simulink model "TosAeb"  (R2021a)
settings: Solver=FixedStepDiscrete  StartTime=0.0  StopTime=inf  FixedStep=0.01  RelTol=1e-3
undefined symbols (not defined in the file or in its folder): ACCModSts, AccSts1, AEB_ObjectMovingOrientation, AEB_ObjectMovingState, CN_BTCActive, CN_CurrentGearR, CN_CurrentGearR_DHT, CN_PTCActive, CN_VDCActive, CN_VSC_EspOff, CN_VSC_FCW_AEB_Error, CN_VSC_FCW_AEB_NoDispay, CN_VSC_FCW_AEB_PerfmDgdtn, CN_VSC_FCW_AEB_TempUnAvlbl, CN_VSC_VehStandstill, CNaccelAvoidMax, CNaccelAvoidMin, CNbrakePedalPress, CNcomfortStrLeftAccelMax, CNcomfortStrLeftAccelMin, CNcomfortStrRightAccelMax, CNcomfortStrRightAccelMin, CNDrvDoorOpen, CNDrvModeEco, CNDrvModeNorm, CNDrvModeOther, CNDrvModeSport, CNDrvSBRreminder, CNNull, CNobjInvalidID, CNPassDoorOpen, CNPassSBRreminder, CNPEBSAccelNegInf, CNPEBSAccelNull, CNPEBSTimeInf, CNPEBSTimeNull, CNSTOPMAX, CNSTOPNULL, CNsubjWidth, CNSwtOFF, CNSwtON, CNTTCMAX, CNTTCNULL, CNVehConfigAEBCycNone, CNVehConfigAEBNone, CNVehConfigAEBPedNone, CNVehConfigFCWNone, CNVehConfigJANone, CollisionSeverity, FALSE, FCWSnvty, JACycObjCalcInfoDefaultParams, JAPedObjCalcInfoDefaultParams, JAVehObjCalcInfoDefaultParams, latCycObjCalcInfoDefaultParams, latPedObjCalcInfoDefaultParams, longObjCalcInfoDefaultParams, ModSts, movingStatus, ObjectType ... (+1112)
libraries: simulink x3031, AEBSFunctionLib x50, Ta_Library x33, AEBSStateMachineLib x23, Mylibrary x12, simulink_extras x7, ObjSelect_lib x3, dspstat3 x2
(part 18 of 102)

== system TosAeb/FcwAebJa_Step_sys/ALG_AEBSystem/ALG_AEBController/AEB_StateMachineCalcApp/VehAEBStateMachine/EBAStateMachine ==
  inputSignal : Inport
  AEBFuncFaultMap : Subsystem
  AEBStateMachine : AEBSStateMachineLib/AEBStateMachine  SystemSampleTime=-1  VariantActivationTime=update diagram  Latency=0  AutoFrameSizeCalculation=off
  ActivationCondCalc : Subsystem
  ActiveStateAborCondCalc : Subsystem
  Bus Selector : BusSelector  OutputSignals=AVHStsActv,vehStateCalcInfo.VSC_FCW_AEB_FctSts.FCW_AEB_VehFctSts,vehStateCalcInfo.VSC_AEBFuncSwtSts.AEB_VehResp,vehStateCalcInfo.VSCVehStandStill
  Bus Selector1 : BusSelector  OutputSignals=VehFuncSuppAborCond.vehEBASuppCond,vehFctStsArbSuppAborCond.brkSuppAborCondArb.EBASuppArb,FuncImpSuppAborCond.brkFuncImpSuppAborCond.brkFuncImpSuppCond.vehEBAFuncImpSuppCond
  Bus Selector2 : BusSelector  OutputSignals=VehFuncSuppAborCond.vehEBAAborCondNoStandstill,vehFctStsArbSuppAborCond.brkSuppAborCondArb.EBAAborArb,FuncImpSuppAborCond.brkFuncImpSuppAborCond.brkFuncImpAborCond.vehEBAFuncImpAborCond,FuncManeuAborCond.vehBrkManeuAborCond
  Bus Selector3 : BusSelector  OutputSignals=VehFuncSuppAborCond.vehEBAAborCondStandstill,vehFctStsArbSuppAborCond.brkSuppAborCondArb.EBAAborArb,FuncImpSuppAborCond.brkFuncImpSuppAborCond.brkFuncImpAborCond.vehEBAFuncImpAborCond,FuncManeuAborCond.vehBrkManeuAborCond
  Constant : Constant  Value=P_AEB_FuncStatus_VehEBAWorkTime
  Constant1 : Constant  Value=P_AEB_FuncStatus_VehEBAStandstillWorkTime
  Constant2 : Constant  Value=Ts
  StandstillAborCondCalc : Subsystem
  Terminator1 : Terminator
  vehEBARawModSts : Outport
  vehEBAModSts : Outport  Port=2
  wires:
  ActiveStateAborCondCalc/1(ActiveStateAborCondFulfilled) -> AEBStateMachine/6
  StandstillAborCondCalc/1(StandstillAborCondFulfilled) -> AEBStateMachine/7
  inputSignal/1 -> Bus Selector3/1, Bus Selector2/1, Bus Selector1/1, Bus Selector/1
  Bus Selector1/3 -> ActivationCondCalc/3(vehEBAFuncImpSuppCond)  "<vehEBAFuncImpSuppCond>"
  Bus Selector1/2 -> ActivationCondCalc/2(EBASuppArb)  "<EBASuppArb>"
  Bus Selector1/1 -> ActivationCondCalc/1(vehEBASuppCond)  "<vehEBASuppCond>"
  Bus Selector2/1 -> ActiveStateAborCondCalc/1(EBAAborCondNoStandstill)  "<vehEBAAborCondNoStandstill>"
  Bus Selector2/2 -> ActiveStateAborCondCalc/2(EBAAborArb)  "<EBAAborArb>"
  Bus Selector2/3 -> ActiveStateAborCondCalc/3(vehEBAFuncImpAborCond)  "<vehEBAFuncImpAborCond>"
  Bus Selector2/4 -> ActiveStateAborCondCalc/4(vehBrkManeuAborCond)  "<vehBrkManeuAborCond>"
  Bus Selector3/1 -> StandstillAborCondCalc/1(EBAAborCondStandstill)  "<vehEBAAborCondStandstill>"
  Bus Selector3/2 -> StandstillAborCondCalc/2(EBAAborArb)  "<EBAAborArb>"
  Bus Selector3/3 -> StandstillAborCondCalc/3(vehEBAFuncImpAborCond)  "<vehEBAFuncImpAborCond>"
  Bus Selector3/4 -> StandstillAborCondCalc/4(vehBrkManeuAborCond)  "<vehBrkManeuAborCond>"
  AEBStateMachine/1 -> vehEBARawModSts/1
  AEBStateMachine/2 -> vehEBAModSts/1  "vehEBAModSts"
  AEBStateMachine/3 -> Terminator1/1
  ActivationCondCalc/1(ActivationCondFulfilled) -> AEBStateMachine/5
  Constant2/1 -> AEBStateMachine/8
  Constant/1 -> AEBStateMachine/9
  Constant1/1 -> AEBStateMachine/10
  Bus Selector/2 -> AEBFuncFaultMap/1(FCW_AEB_VehFctSts)  "<FCW_AEB_VehFctSts>"
  Bus Selector/3 -> AEBStateMachine/3  "<AEB_VehResp>"
  Bus Selector/1 -> AEBStateMachine/1  "<AVHStsActv>"
  Bus Selector/4 -> AEBStateMachine/4  "<VSCVehStandStill>"
  AEBFuncFaultMap/1(Fault) -> AEBStateMachine/2

== system TosAeb/FcwAebJa_Step_sys/ALG_AEBSystem/ALG_AEBController/AEB_StateMachineCalcApp/VehAEBStateMachine/LatentWarningStateMachine ==
  inputSignal : Inport
  AEBFuncFaultMap : Subsystem
  AEBStateMachine : AEBSStateMachineLib/AEBStateMachine  SystemSampleTime=-1  VariantActivationTime=update diagram  Latency=0  AutoFrameSizeCalculation=off
  ActivationCondCalc : Subsystem
  ActiveStateAborCondCalc : Subsystem
  Bus Selector : BusSelector  OutputSignals=vehStateCalcInfo.VSC_FCW_AEB_FctSts.FCW_AEB_VehFctSts,vehStateCalcInfo.VSC_AEBFuncSwtSts.AEB_VehResp
  Bus Selector1 : BusSelector  OutputSignals=VehFuncSuppAborCond.vehLatentWarningSuppCond,vehFctStsArbSuppAborCond.warningSuppAborCond.latentWarningSuppArb,FuncImpSuppAborCond.warningFuncImpSuppAborCond.warningFuncImpSuppCond.vehLatentWarningFuncImpSuppCond
  Bus Selector2 : BusSelector  OutputSignals=VehFuncSuppAborCond.vehLatentWarningAborCond,vehFctStsArbSuppAborCond.warningSuppAborCond.latentWarningAborArb
  Constant : Constant  Value=P_AEB_FuncStatus_VehLatentWarningTime
  Constant1 : Constant  Value=P_AEB_FuncStatus_VehLatentWarningStandstillWorkTime
  Constant2 : Constant  Value=Ts
  Constant3 : Constant  Value=FALSE
  Constant4 : Constant  Value=FALSE
  Constant5 : Constant  Value=FALSE
  Terminator1 : Terminator
  vehLatentWarningRawModSts : Outport
  vehLatentWarningModSts : Outport  Port=2
  wires:
  ActiveStateAborCondCalc/1(ActiveStateAborCondFulfilled) -> AEBStateMachine/6
  ActivationCondCalc/1(ActivationCondFulfilled) -> AEBStateMachine/5
  inputSignal/1 -> Bus Selector/1, Bus Selector2/1, Bus Selector1/1
  Bus Selector1/2 -> ActivationCondCalc/2(latentWarningSuppArb)  "<latentWarningSuppArb>"
  Bus Selector1/3 -> ActivationCondCalc/3(vehLatentWarningFuncImpSuppCond)  "<vehLatentWarningFuncImpSuppCond>"
  Bus Selector1/1 -> ActivationCondCalc/1(vehLatentWarningSuppCond)  "<vehLatentWarningSuppCond>"
  Bus Selector2/1 -> ActiveStateAborCondCalc/1(vehLatentWarningAborCond)  "<vehLatentWarningAborCond>"
  Bus Selector2/2 -> ActiveStateAborCondCalc/2(latentWarningAbor)  "<latentWarningAborArb>"
  AEBStateMachine/2 -> vehLatentWarningModSts/1  "vehLatentWarningModSts"
  AEBStateMachine/1 -> vehLatentWarningRawModSts/1
  AEBStateMachine/3 -> Terminator1/1
  Constant3/1 -> AEBStateMachine/7
  Constant2/1 -> AEBStateMachine/8
  Constant/1 -> AEBStateMachine/9
  Constant1/1 -> AEBStateMachine/10
  Constant5/1 -> AEBStateMachine/1
  Constant4/1 -> AEBStateMachine/4
  Bus Selector/1 -> AEBFuncFaultMap/1(FCW_AEB_VehFctSts)  "<FCW_AEB_VehFctSts>"
  Bus Selector/2 -> AEBStateMachine/3  "<AEB_VehResp>"
  AEBFuncFaultMap/1(Fault) -> AEBStateMachine/2

== system TosAeb/FcwAebJa_Step_sys/ALG_AEBSystem/ALG_AEBController/AEB_StateMachineCalcApp/VehAEBStateMachine/PreWarningStateMachine ==
  inputSignal : Inport
  AEBFuncFaultMap : Subsystem
  AEBStateMachine : AEBSStateMachineLib/AEBStateMachine  SystemSampleTime=-1  VariantActivationTime=update diagram  Latency=0  AutoFrameSizeCalculation=off
  ActivationCondCalc : Subsystem
  ActiveStateAborCondCalc : Subsystem
  Bus Selector : BusSelector  OutputSignals=vehStateCalcInfo.VSC_FCW_AEB_FctSts.FCW_AEB_VehFctSts,vehStateCalcInfo.VSC_AEBFuncSwtSts.AEB_VehResp
  Bus Selector1 : BusSelector  OutputSignals=VehFuncSuppAborCond.vehPreWarningSuppCond,vehFctStsArbSuppAborCond.warningSuppAborCond.preWarningSuppArb,FuncImpSuppAborCond.warningFuncImpSuppAborCond.warningFuncImpSuppCond.vehPreWarningFuncImpSuppCond
  Bus Selector2 : BusSelector  OutputSignals=VehFuncSuppAborCond.vehPreWarningAborCond,vehFctStsArbSuppAborCond.warningSuppAborCond.preWarningAborArb,FuncImpSuppAborCond.warningFuncImpSuppAborCond.warningFuncImpAborCond.vehPreWarningFuncImpAborCond
  Constant : Constant  Value=P_AEB_FuncStatus_VehPreWarningWorkTime
  Constant1 : Constant  Value=P_AEB_FuncStatus_VehPreWarningStandstillWorkTime
  Constant2 : Constant  Value=Ts
  Constant3 : Constant  Value=FALSE
  Constant4 : Constant  Value=FALSE
  Constant5 : Constant  Value=FALSE
  Terminator1 : Terminator
  vehPreWarningRawModSts : Outport
  vehPreWarningModSts : Outport  Port=2
  wires:
  AEBStateMachine/2 -> vehPreWarningModSts/1  "vehPreWarningModSts"
  inputSignal/1 -> Bus Selector1/1, Bus Selector2/1, Bus Selector/1
  Bus Selector1/2 -> ActivationCondCalc/2(preWarningSuppArb)  "<preWarningSuppArb>"
  Bus Selector1/3 -> ActivationCondCalc/3(vehPreWarningFuncImpSuppCond)  "<vehPreWarningFuncImpSuppCond>"
  Bus Selector1/1 -> ActivationCondCalc/1(vehPreWarningSuppCond)  "<vehPreWarningSuppCond>"
  Bus Selector2/1 -> ActiveStateAborCondCalc/1(vehPreWarningAborCond)  "<vehPreWarningAborCond>"
  Bus Selector2/2 -> ActiveStateAborCondCalc/2(preWarningSuppArb)  "<preWarningAborArb>"
  Bus Selector2/3 -> ActiveStateAborCondCalc/3(vehPreWarningFuncImpAborCond)  "<vehPreWarningFuncImpAborCond>"
  AEBStateMachine/1 -> vehPreWarningRawModSts/1
  AEBStateMachine/3 -> Terminator1/1
  Constant5/1 -> AEBStateMachine/1
  Bus Selector/1 -> AEBFuncFaultMap/1(FCW_AEB_VehFctSts)  "<FCW_AEB_VehFctSts>"
  Bus Selector/2 -> AEBStateMachine/3  "<AEB_VehResp>"
  Constant4/1 -> AEBStateMachine/4
  ActivationCondCalc/1(vehPreWarningActivationCondFulfilled) -> AEBStateMachine/5
  ActiveStateAborCondCalc/1(vehPreWarningAborCondFulfilled) -> AEBStateMachine/6
  Constant3/1 -> AEBStateMachine/7
  Constant2/1 -> AEBStateMachine/8
  Constant/1 -> AEBStateMachine/9
  Constant1/1 -> AEBStateMachine/10
  AEBFuncFaultMap/1(Fault) -> AEBStateMachine/2

== system TosAeb/FcwAebJa_Step_sys/ALG_AEBSystem/ALG_AEBController/AEB_StateMachineCalcApp/VehAEBStateMachine/PrefillStateMachine ==
  inputSignal : Inport
  AEBFuncFaultMap : Subsystem
  AEBStateMachine : AEBSStateMachineLib/AEBStateMachine  SystemSampleTime=-1  VariantActivationTime=update diagram  Latency=0  AutoFrameSizeCalculation=off
  ActivationCondCalc : Subsystem
  ActiveStateAborCondCalc : Subsystem
  Bus Selector : BusSelector  OutputSignals=vehStateCalcInfo.VSC_FCW_AEB_FctSts.FCW_AEB_VehFctSts,vehStateCalcInfo.VSC_AEBFuncSwtSts.AEB_VehResp
  Bus Selector1 : BusSelector  OutputSignals=VehFuncSuppAborCond.vehPrefillSuppCond,FuncImpSuppAborCond.brkReadyFuncImpSuppAborCond.brkReadyFuncImpSuppCond.vehPrefillFuncImpSuppCond,vehFctStsArbSuppAborCond.brkReadySuppAborCond.prefillBrkReadySuppArb
  Bus Selector2 : BusSelector  OutputSignals=VehFuncSuppAborCond.vehPrefillAborCond,vehFctStsArbSuppAborCond.brkReadySuppAborCond.prefillBrkReadyAborArb,FuncImpSuppAborCond.brkReadyFuncImpSuppAborCond.brkReadyFuncImpAborCond.vehPrefillFuncImpAborCond,FuncManeuAborCond.ABPFuncManeuAborCond
  Constant : Constant  Value=P_AEB_FuncStatus_VehPrefillWorkTime
  Constant1 : Constant  Value=P_AEB_FuncStatus_VehPrefillStandstillWorkTime
  Constant2 : Constant  Value=Ts
  Constant3 : Constant  Value=FALSE
  Constant4 : Constant  Value=FALSE
  Constant5 : Constant  Value=FALSE
  Terminator1 : Terminator
  vehPrefillRawModSts : Outport
  vehPrefillModSts : Outport  Port=2
  wires:
  inputSignal/1 -> Bus Selector2/1, Bus Selector1/1, Bus Selector/1
  Bus Selector1/2 -> ActivationCondCalc/2(vehPrefillFuncImpSuppCond)  "<vehPrefillFuncImpSuppCond>"
  Bus Selector1/3 -> ActivationCondCalc/3(vehPrefillBrkReadySuppArb)  "<prefillBrkReadySuppArb>"
  Bus Selector1/1 -> ActivationCondCalc/1(vehPrefillSuppCond)  "<vehPrefillSuppCond>"
  Bus Selector2/1 -> ActiveStateAborCondCalc/1(vehPrefillAborCond)  "<vehPrefillAborCond>"
  Bus Selector2/2 -> ActiveStateAborCondCalc/2(prefillBrkReadyAborArb)  "<prefillBrkReadyAborArb>"
  Bus Selector2/3 -> ActiveStateAborCondCalc/3(vehPrefillFuncImpAborCond)  "<vehPrefillFuncImpAborCond>"
  Bus Selector2/4 -> ActiveStateAborCondCalc/4(brkImpOpcAborCond)  "<ABPFuncManeuAborCond>"
  AEBStateMachine/3 -> Terminator1/1
  AEBStateMachine/1 -> vehPrefillRawModSts/1
  AEBStateMachine/2 -> vehPrefillModSts/1  "vehPrefillModSts"
  Constant5/1 -> AEBStateMachine/1
  Bus Selector/1 -> AEBFuncFaultMap/1(FCW_AEB_VehFctSts)  "<FCW_AEB_VehFctSts>"
  Bus Selector/2 -> AEBStateMachine/3  "<AEB_VehResp>"
  Constant4/1 -> AEBStateMachine/4
  ActivationCondCalc/1(ActivationCondFulfilled) -> AEBStateMachine/5
  ActiveStateAborCondCalc/1(ActiveStateAborCondFulfilled) -> AEBStateMachine/6
  Constant3/1 -> AEBStateMachine/7
  Constant2/1 -> AEBStateMachine/8
  Constant/1 -> AEBStateMachine/9
  Constant1/1 -> AEBStateMachine/10
  AEBFuncFaultMap/1(Fault) -> AEBStateMachine/2

== system TosAeb/FcwAebJa_Step_sys/ALG_AEBSystem/ALG_AEBController/AEB_StateMachineCalcApp/VehFCTModStsArb/BrkArb ==
  vehAEBModSts : Inport
  BrkAborArb : Subsystem
  BrkActvArb : Subsystem
  BrkSuppArb : Subsystem
  Bus Creator : BusCreator  Inputs=6
  WarningModStsArbtrtn : Subsystem
  brkActvCond : Outport
  AEBpartModStsArb : Outport  Port=2
  AEBfullModStsArb : Outport  Port=3
  EBAModStsArb : Outport  Port=4
  brkSuppAborCondArb : Outport  Port=5
  wires:
  [AEBfullModStsArb] -> AEBfullModStsArb/1
  [AEBpartModStsArb] -> AEBpartModStsArb/1
  WarningModStsArbtrtn/3(EBAModStsArb) -> EBAModStsArb/1, BrkActvArb/3(EBAModStsArbtrtn)
  BrkAborArb/3(EBAAborArb) -> [EBAAborArb], Bus Creator/6  "EBAAborArb"
  [AEBfullAborArb] -> WarningModStsArbtrtn/3(AEBfullAborArb)
  BrkSuppArb/2(AEBfullSuppArb) -> Bus Creator/2  "AEBfullSuppArb"
  BrkActvArb/1(brkActvCond) -> brkActvCond/1
  BrkAborArb/2(AEBfullAborArb) -> [AEBfullAborArb], Bus Creator/5  "AEBfullAborArb"
  vehAEBModSts/1 -> BrkAborArb/1(vehAEBModSts), BrkSuppArb/1(vehAEBModSts), WarningModStsArbtrtn/1(vehAEBModSts)
  BrkSuppArb/3(EBASuppArb) -> Bus Creator/3  "EBASuppArb"
  Bus Creator/1 -> brkSuppAborCondArb/1
  BrkAborArb/1(AEBpartAborArb) -> [AEBpartAborArb], Bus Creator/4  "AEBpartAborArb"
  [AEBpartAborArb] -> WarningModStsArbtrtn/2(AEBpartAborArb)
  [EBAAborArb] -> WarningModStsArbtrtn/4(EBAAborArb)
  BrkSuppArb/1(AEBpartSuppArb) -> Bus Creator/1  "AEBpartSuppArb"
  WarningModStsArbtrtn/1(AEBpartModStsArb) -> [AEBpartModStsArb], BrkActvArb/1(AEBpartModStsArbtrtn)
  WarningModStsArbtrtn/2(AEBfullModStsArb) -> [AEBfullModStsArb], BrkActvArb/2(AEBfullModStsArbtrtn)

== system TosAeb/FcwAebJa_Step_sys/ALG_AEBSystem/ALG_AEBController/AEB_StateMachineCalcApp/VehFCTModStsArb/BrkReadyArb ==
  VehAEBModSts : Inport
  brkActvCond : Inport  Port=2
  BrkReadyAborArb : Subsystem
  BrkReadyActvArb : Subsystem
  BrkReadyModStsArb : Subsystem
  BrkReadySuppArb : Subsystem
  Bus Creator : BusCreator  Inputs=4
  brkReadyActvCond : Outport
  prefillModStsArb : Outport  Port=2
  ABAModStsArb : Outport  Port=3
  brkReadySuppAborCond : Outport  Port=4
  wires:
  VehAEBModSts/1 -> BrkReadyModStsArb/1(vehAEBModSts)
  brkActvCond/1 -> BrkReadyAborArb/1(brkActvCond), BrkReadySuppArb/1(brkActvCond)
  BrkReadyAborArb/1(prefillBrkReadyAborArb) -> Bus Creator/3, [prefillBrkReadyAbor]  "prefillBrkReadyAborArb"
  BrkReadyAborArb/2(ABABrkReadyAborArb) -> [ABABrkReadyAbor], Bus Creator/4  "ABABrkReadyAborArb"
  Bus Creator/1 -> brkReadySuppAborCond/1
  [ABABrkReadyAbor] -> BrkReadyModStsArb/3(ABABrkReadyAbor)
  [prefillBrkReadyAbor] -> BrkReadyModStsArb/2(prefillBrkReadyAbor)
  BrkReadyActvArb/1(brkReadyActv) -> brkReadyActvCond/1
  BrkReadySuppArb/2(ABABrkReadySuppArb) -> Bus Creator/2  "ABABrkReadySuppArb"
  BrkReadySuppArb/1(prefillBrkReadySuppArb) -> Bus Creator/1  "prefillBrkReadySuppArb"
  BrkReadyModStsArb/1(prefillModStsArb) -> prefillModStsArb/1, BrkReadyActvArb/1(prefillModStsArb)
  BrkReadyModStsArb/2(ABAModStsArb) -> ABAModStsArb/1, BrkReadyActvArb/2(ABAModStsArb)

== system TosAeb/FcwAebJa_Step_sys/ALG_AEBSystem/ALG_AEBController/AEB_StateMachineCalcApp/VehFCTModStsArb/WarningArb ==
  vehAEBModSts : Inport
  brkReadyActvCond : Inport  Port=2
  brkActvCond : Inport  Port=3
  BrkReadyAborArb : Subsystem
  Bus Creator : BusCreator  Inputs=6
  Terminator : Terminator
  WarningActvArbtrtn : Subsystem
  WarningModStsArbtrtn : Subsystem
  WarningSuppArb : Subsystem
  latentWarningModStsArb : Outport
  preWarningModStsArb : Outport  Port=2
  acuteWarningModStsArb : Outport  Port=3
  warningSuppAborCond : Outport  Port=4
  wires:
  Bus Creator/1 -> warningSuppAborCond/1
  vehAEBModSts/1 -> WarningModStsArbtrtn/1(vehAEBModSts), WarningSuppArb/1(vehAEBModSts), BrkReadyAborArb/3(vehAEBModSts)
  brkActvCond/1 -> WarningSuppArb/3(brkActvCond), BrkReadyAborArb/1(brkActvCond)
  brkReadyActvCond/1 -> WarningSuppArb/2(brkReadyActvCond), BrkReadyAborArb/2(brkReadyActvCond)
  WarningSuppArb/3(acutewarningSuppArb) -> Bus Creator/3  "acuteWarningSuppArb"
  WarningSuppArb/2(prewarningSuppArb) -> Bus Creator/2  "preWarningSuppArb"
  WarningSuppArb/1(latentWarningSuppArb) -> Bus Creator/1  "latentWarningSuppArb"
  BrkReadyAborArb/1(latentWarningAborArb) -> [latentWarningAborArb], Bus Creator/4  "latentWarningAborArb"
  BrkReadyAborArb/2(preWarningAborArb) -> [preWarningAborArb], Bus Creator/5  "preWarningAborArb"
  WarningModStsArbtrtn/1(latentWarningModStsArb) -> WarningActvArbtrtn/1(latentWarningModStsArb), [latentWarningModStsArb]
  WarningModStsArbtrtn/2(preWarningModStsArb) -> [preWarningModStsArb], WarningActvArbtrtn/2(preWarningModStsArb)
  WarningModStsArbtrtn/3(acuteWarningModStsArb) -> acuteWarningModStsArb/1, WarningActvArbtrtn/3(acuteWarningModStsArb)
  BrkReadyAborArb/3(acuteWarningAborArb) -> [acuteWarningAborArb], Bus Creator/6  "acuteWarningAborArb"
  [latentWarningAborArb] -> WarningModStsArbtrtn/2(latentWarningAborArb)
  [acuteWarningAborArb] -> WarningModStsArbtrtn/4(acuteWarningAborArb)
  [preWarningAborArb] -> WarningModStsArbtrtn/3(preWarningAborArb)
  [latentWarningModStsArb] -> latentWarningModStsArb/1
  [preWarningModStsArb] -> preWarningModStsArb/1
  WarningActvArbtrtn/1(WarningActvCond) -> Terminator/1

== system TosAeb/FcwAebJa_Step_sys/ALG_AEBSystem/ALG_AEBController/AEB_VehicleStateCalcApp/CycSuppAborCondtionCalc/CycAEBfullSuppAborConditonCalc ==
  VSCAEB_PedResp : Inport
  FCW_AEB_PedFctSts : Inport  Port=2
  vehUnstableStateFlg : Inport  Port=3
  vehAssociatedSysStateSuppCond : Inport  Port=4
  "vehConfigAEBCyc " : Inport  Port=5
  blockTimeBrakeReady : Inport  Port=6
  Bus Creator : BusCreator  Inputs=6
  Bus Selector : BusSelector  OutputSignals=AEB_PedResp,FCW_AEB_PedFctSts,vehUnstableSts,vehAssociatedSysStsSuppFlg,VehicleConfigAEBCyc,blockTimeBrake
  Bus Selector1 : BusSelector  OutputSignals=AEB_PedResp,FCW_AEB_PedFctSts,vehAssociatedSysStsSuppFlg,vehUnstableSts
  VehStateCalcCycAEBfullAborCalc : Subsystem
  VehStateCalcCycAEBfullSuppCalc : Subsystem
  VSCCycAEBfullSuppCond : Outport
  VSCCycAEBfullAborCond : Outport  Port=2
  wires:
  VehStateCalcCycAEBfullAborCalc/1(VSCCycAEBfullAborCond) -> VSCCycAEBfullAborCond/1
  VehStateCalcCycAEBfullSuppCalc/1(VSCCycAEBfullSuppCond) -> VSCCycAEBfullSuppCond/1
  vehUnstableStateFlg/1 -> Bus Creator/3
  FCW_AEB_PedFctSts/1 -> Bus Creator/2
  vehAssociatedSysStateSuppCond/1 -> Bus Creator/4
  VSCAEB_PedResp/1 -> Bus Creator/1
  "vehConfigAEBCyc "/1 -> Bus Creator/5
  blockTimeBrakeReady/1 -> Bus Creator/6
  Bus Creator/1 -> Bus Selector/1, Bus Selector1/1
  Bus Selector/4 -> VehStateCalcCycAEBfullSuppCalc/4(vehAssociatedSysStateSuppCond)  "<vehAssociatedSysStsSuppFlg>"
  Bus Selector/3 -> VehStateCalcCycAEBfullSuppCalc/3(vehUnstableStateFlg)  "<vehUnstableSts>"
  Bus Selector/5 -> VehStateCalcCycAEBfullSuppCalc/5("vehConfigAEBCyc ")  "<VehicleConfigAEBCyc>"
  Bus Selector/2 -> VehStateCalcCycAEBfullSuppCalc/2(FCW_AEB_PedFctSts)  "<FCW_AEB_PedFctSts>"
  Bus Selector/6 -> VehStateCalcCycAEBfullSuppCalc/6(blockTimeBrakeReady)  "<blockTimeBrake>"
  Bus Selector/1 -> VehStateCalcCycAEBfullSuppCalc/1(VSCAEB_PedResp)  "<AEB_PedResp>"
  Bus Selector1/1 -> VehStateCalcCycAEBfullAborCalc/1(VSCAEB_PedResp)  "<AEB_PedResp>"
  Bus Selector1/2 -> VehStateCalcCycAEBfullAborCalc/2(FCW_AEB_PedFctSts)  "<FCW_AEB_PedFctSts>"
  Bus Selector1/3 -> VehStateCalcCycAEBfullAborCalc/3(vehAssociatedSysStateSuppCond)  "<vehAssociatedSysStsSuppFlg>"
  Bus Selector1/4 -> VehStateCalcCycAEBfullAborCalc/4(vehUnstableStateFlg)  "<vehUnstableSts>"

== system TosAeb/FcwAebJa_Step_sys/ALG_AEBSystem/ALG_AEBController/AEB_VehicleStateCalcApp/CycSuppAborCondtionCalc/CycAEBpartSuppAborConditonCalc ==
  VSCAEB_PedResp : Inport
  FCW_AEB_PedFctSts : Inport  Port=2
  vehUnstableStateFlg : Inport  Port=3
  vehAssociatedSysStateSuppCond : Inport  Port=4
  "vehConfigAEBCyc " : Inport  Port=5
  blockTimeBrake : Inport  Port=6
  Bus Creator : BusCreator  Inputs=6
  Bus Selector : BusSelector  OutputSignals=AEB_PedResp,FCW_AEB_PedFctSts,vehUnstableSts,vehAssociatedSysStsSuppFlg,VehicleConfigAEBCyc,blockTimeBrake
  Bus Selector1 : BusSelector  OutputSignals=AEB_PedResp,FCW_AEB_PedFctSts,vehAssociatedSysStsSuppFlg,vehUnstableSts
  VehStateCalcCycAEBpartAborCalc : Subsystem
  VehStateCalcCycAEBpartSuppCalc : Subsystem
  VSCAEBpartSuppCond : Outport
  VSCAEBpartAborCond : Outport  Port=2
  wires:
  VehStateCalcCycAEBpartAborCalc/1(VSCAEBpartAborCond) -> VSCAEBpartAborCond/1
  VehStateCalcCycAEBpartSuppCalc/1(VSCAEBpartSuppCond) -> VSCAEBpartSuppCond/1
  "vehConfigAEBCyc "/1 -> Bus Creator/5
  vehAssociatedSysStateSuppCond/1 -> Bus Creator/4
  blockTimeBrake/1 -> Bus Creator/6
  vehUnstableStateFlg/1 -> Bus Creator/3
  FCW_AEB_PedFctSts/1 -> Bus Creator/2
  VSCAEB_PedResp/1 -> Bus Creator/1
  Bus Creator/1 -> Bus Selector/1, Bus Selector1/1
  Bus Selector/1 -> VehStateCalcCycAEBpartSuppCalc/1(AEB_PedResp)  "<AEB_PedResp>"
  Bus Selector/2 -> VehStateCalcCycAEBpartSuppCalc/2(FCW_AEB_PedFctSts)  "<FCW_AEB_PedFctSts>"
  Bus Selector/3 -> VehStateCalcCycAEBpartSuppCalc/3(vehUnstableStateFlg)  "<vehUnstableSts>"
  Bus Selector/4 -> VehStateCalcCycAEBpartSuppCalc/4(vehAssociatedSysStateSuppCond)  "<vehAssociatedSysStsSuppFlg>"
  Bus Selector/5 -> VehStateCalcCycAEBpartSuppCalc/5("vehConfigAEBCyc ")  "<VehicleConfigAEBCyc>"
  Bus Selector/6 -> VehStateCalcCycAEBpartSuppCalc/6(blockTimeBrake)  "<blockTimeBrake>"
  Bus Selector1/2 -> VehStateCalcCycAEBpartAborCalc/2(FCW_AEB_PedFctSts)  "<FCW_AEB_PedFctSts>"
  Bus Selector1/1 -> VehStateCalcCycAEBpartAborCalc/1(AEB_PedResp)  "<AEB_PedResp>"
  Bus Selector1/3 -> VehStateCalcCycAEBpartAborCalc/3(vehAssociatedSysStateSuppCond)  "<vehAssociatedSysStsSuppFlg>"
  Bus Selector1/4 -> VehStateCalcCycAEBpartAborCalc/4(vehUnstableStateFlg)  "<vehUnstableSts>"

== system TosAeb/FcwAebJa_Step_sys/ALG_AEBSystem/ALG_AEBController/AEB_VehicleStateCalcApp/CycSuppAborCondtionCalc/CycPrefillSuppAborConditonCalc ==
  AEB_PedResp : Inport
  FCW_AEB_PedFctSts : Inport  Port=2
  vehUnstableStateFlg : Inport  Port=3
  vehAssociatedSysStateSuppCond : Inport  Port=4
  "vehConfigAEBCyc " : Inport  Port=5
  blockTimeBrakeReady : Inport  Port=6
  Bus Creator : BusCreator  Inputs=6
  Bus Selector : BusSelector  OutputSignals=AEB_PedResp,FCW_AEB_PedFctSts,vehUnstableSts,vehAssociatedSysStsSuppFlg,VehicleConfigAEBCyc,blockTimeBrakeReady
  Bus Selector1 : BusSelector  OutputSignals=AEB_PedResp,FCW_AEB_PedFctSts,vehUnstableSts,vehAssociatedSysStsSuppFlg
  VehStateCalcPrefillAborCalc : Subsystem
  VehStateCalcPrefillSuppCalc : Subsystem
  VSCCycPrefillSuppCond : Outport
  VSCCycPrefillAborCond : Outport  Port=2
  wires:
  VehStateCalcPrefillAborCalc/1(VSCCycPrefillAborCond) -> VSCCycPrefillAborCond/1
  VehStateCalcPrefillSuppCalc/1(VSCCycPrefillSuppCond) -> VSCCycPrefillSuppCond/1
  vehUnstableStateFlg/1 -> Bus Creator/3
  FCW_AEB_PedFctSts/1 -> Bus Creator/2
  vehAssociatedSysStateSuppCond/1 -> Bus Creator/4
  AEB_PedResp/1 -> Bus Creator/1
  "vehConfigAEBCyc "/1 -> Bus Creator/5
  blockTimeBrakeReady/1 -> Bus Creator/6
  Bus Creator/1 -> Bus Selector/1, Bus Selector1/1
  Bus Selector/4 -> VehStateCalcPrefillSuppCalc/4(vehAssociatedSysStateSuppCond)  "<vehAssociatedSysStsSuppFlg>"
  Bus Selector/3 -> VehStateCalcPrefillSuppCalc/3(vehUnstableStateFlg)  "<vehUnstableSts>"
  Bus Selector/5 -> VehStateCalcPrefillSuppCalc/5("vehConfigAEBCyc ")  "<VehicleConfigAEBCyc>"
  Bus Selector/2 -> VehStateCalcPrefillSuppCalc/2(FCW_AEB_PedFctSts)  "<FCW_AEB_PedFctSts>"
  Bus Selector/6 -> VehStateCalcPrefillSuppCalc/6(blockTimeBrakeReady)  "<blockTimeBrakeReady>"
  Bus Selector/1 -> VehStateCalcPrefillSuppCalc/1(VSCAEB_PedResp)  "<AEB_PedResp>"
  Bus Selector1/1 -> VehStateCalcPrefillAborCalc/1(AEB_PedResp)  "<AEB_PedResp>"
  Bus Selector1/2 -> VehStateCalcPrefillAborCalc/2(FCW_AEB_PedFctSts)  "<FCW_AEB_PedFctSts>"
  Bus Selector1/3 -> VehStateCalcPrefillAborCalc/3(vehUnstableStateFlg)  "<vehUnstableSts>"
  Bus Selector1/4 -> VehStateCalcPrefillAborCalc/4(vehAssociatedSysStateSuppCond)  "<vehAssociatedSysStsSuppFlg>"

== system TosAeb/FcwAebJa_Step_sys/ALG_AEBSystem/ALG_AEBController/AEB_VehicleStateCalcApp/FuncSwtStateJudge/Subsystem3 ==
  note: 0x00:Normal
      | 0x01:High sensitivity
      | 0x02:Low sensitivity
      | 0x03:Reserved
  ALG_FCWLevelReq : Inport
  Constant2 : Constant
  Constant5 : Constant  Value=0
  Constant6 : Constant  Value=2
  Multiport Switch1 : MultiPortSwitch  DataPortOrder=Specify indices  DataPortIndices={1,3}  DataPortForDefault=Additional data port  DiagnosticForDefault=None
  FCWLevelSts : Outport
  wires:
  Constant6/1 -> Multiport Switch1/2
  Constant5/1 -> Multiport Switch1/4
  ALG_FCWLevelReq/1 -> Multiport Switch1/1
  Constant2/1 -> Multiport Switch1/3
  Multiport Switch1/1 -> FCWLevelSts/1  "FCWLevelSts"

== system TosAeb/FcwAebJa_Step_sys/ALG_AEBSystem/ALG_AEBController/AEB_VehicleStateCalcApp/JaSuppAborCondtionCalc/JACycAEBSuppAborConditonCalc ==
  AEB_PedResp : Inport
  FCW_AEB_JAFctSts : Inport  Port=2
  vehUnstableStateFlg : Inport  Port=3
  vehAssociatedSysStateSuppCond : Inport  Port=4
  "VehicleConfigAEBPed " : Inport  Port=5
  blockTimeBrake : Inport  Port=6
  Bus Creator : BusCreator  Inputs=6
  Bus Selector : BusSelector  OutputSignals=AEB_PedResp,FCW_AEB_JAFctSts,vehUnstableStateFlg,vehAssociatedSysStateSuppCond,VehicleConfigAEBPed,blockTimeBrake
  Bus Selector1 : BusSelector  OutputSignals=AEB_PedResp,FCW_AEB_JAFctSts,vehAssociatedSysStateSuppCond,vehUnstableStateFlg
  VehStateCalcJAAEBAborCalc : Subsystem
  VehStateCalcJAAEBSuppCalc : Subsystem
  VSC_JACycAEBfullSuppCond : Outport
  VSC_JACycAEBfullAborCond : Outport  Port=2
  wires:
  Bus Selector1/4 -> VehStateCalcJAAEBAborCalc/4(vehUnstableStateFlg)  "<vehUnstableStateFlg>"
  Bus Selector1/3 -> VehStateCalcJAAEBAborCalc/3(vehAssociatedSysStateSuppCond)  "<vehAssociatedSysStateSuppCond>"
  Bus Selector1/2 -> VehStateCalcJAAEBAborCalc/2(FCW_AEB_JAFctSts)  "<FCW_AEB_JAFctSts>"
  Bus Selector1/1 -> VehStateCalcJAAEBAborCalc/1(AEB_PedResp)  "<AEB_PedResp>"
  Bus Selector/1 -> VehStateCalcJAAEBSuppCalc/1(AEB_PedResp)  "<AEB_PedResp>"
  Bus Selector/6 -> VehStateCalcJAAEBSuppCalc/6(blockTimeBrake)  "<blockTimeBrake>"
  Bus Selector/2 -> VehStateCalcJAAEBSuppCalc/2(FCW_AEB_JAFctSts)  "<FCW_AEB_JAFctSts>"
  Bus Selector/5 -> VehStateCalcJAAEBSuppCalc/5("VehicleConfigAEBPed ")  "<VehicleConfigAEBPed>"
  Bus Selector/3 -> VehStateCalcJAAEBSuppCalc/3(vehUnstableStateFlg)  "<vehUnstableStateFlg>"
  Bus Selector/4 -> VehStateCalcJAAEBSuppCalc/4(vehAssociatedSysStateSuppCond)  "<vehAssociatedSysStateSuppCond>"
  Bus Creator/1 -> Bus Selector/1, Bus Selector1/1
  AEB_PedResp/1 -> Bus Creator/1  "AEB_PedResp"
  FCW_AEB_JAFctSts/1 -> Bus Creator/2  "FCW_AEB_JAFctSts"
  vehUnstableStateFlg/1 -> Bus Creator/3  "vehUnstableStateFlg"
  vehAssociatedSysStateSuppCond/1 -> Bus Creator/4  "vehAssociatedSysStateSuppCond"
  "VehicleConfigAEBPed "/1 -> Bus Creator/5  "VehicleConfigAEBPed"
  blockTimeBrake/1 -> Bus Creator/6  "blockTimeBrake"
  VehStateCalcJAAEBSuppCalc/1(VSC_JACycAEBfullSuppCond) -> VSC_JACycAEBfullSuppCond/1
  VehStateCalcJAAEBAborCalc/1(VSC_JACycAEBfullAborCond) -> VSC_JACycAEBfullAborCond/1

== system TosAeb/FcwAebJa_Step_sys/ALG_AEBSystem/ALG_AEBController/AEB_VehicleStateCalcApp/JaSuppAborCondtionCalc/JACycPrefillSuppAborConditonCalc ==
  AEB_PedResp : Inport
  FCW_AEB_JAFctSts : Inport  Port=2
  vehUnstableStateFlg : Inport  Port=3
  vehAssociatedSysStateSuppCond : Inport  Port=4
  "VehicleConfigAEBPed " : Inport  Port=5
  blockTimeBrakeReady : Inport  Port=6
  Bus Creator1 : BusCreator  Inputs=6
  Bus Selector : BusSelector  OutputSignals=AEB_PedResp,FCW_AEB_JAFctSts,vehUnstableStateFlg,vehAssociatedSysStateSuppCond,VehicleConfigAEBPed,blockTimeBrakeReady
  Bus Selector1 : BusSelector  OutputSignals=AEB_PedResp,FCW_AEB_JAFctSts,vehAssociatedSysStateSuppCond,vehUnstableStateFlg
  VehStateCalcJAPrefillAborCalc : Subsystem
  VehStateCalcJAPrefillSuppCalc : Subsystem
  VSC_JACycPrefillSuppCond : Outport
  VSC_JACycPrefillAborCond : Outport  Port=2
  wires:
  Bus Selector1/4 -> VehStateCalcJAPrefillAborCalc/4(vehUnstableStateFlg)  "<vehUnstableStateFlg>"
  Bus Selector1/3 -> VehStateCalcJAPrefillAborCalc/3(vehAssociatedSysStateSuppCond)  "<vehAssociatedSysStateSuppCond>"
  Bus Selector1/2 -> VehStateCalcJAPrefillAborCalc/2(FCW_AEB_JAFctSts)  "<FCW_AEB_JAFctSts>"
  Bus Selector1/1 -> VehStateCalcJAPrefillAborCalc/1(AEB_PedResp)  "<AEB_PedResp>"
  Bus Selector/1 -> VehStateCalcJAPrefillSuppCalc/1(AEB_PedResp)  "<AEB_PedResp>"
  Bus Selector/6 -> VehStateCalcJAPrefillSuppCalc/6(blockTimeBrakeReady)  "<blockTimeBrakeReady>"
  Bus Selector/2 -> VehStateCalcJAPrefillSuppCalc/2(FCW_AEB_JAFctSts)  "<FCW_AEB_JAFctSts>"
  Bus Selector/5 -> VehStateCalcJAPrefillSuppCalc/5("VehicleConfigAEBPed ")  "<VehicleConfigAEBPed>"
  Bus Selector/3 -> VehStateCalcJAPrefillSuppCalc/3(vehUnstableStateFlg)  "<vehUnstableStateFlg>"
  Bus Selector/4 -> VehStateCalcJAPrefillSuppCalc/4(vehAssociatedSysStateSuppCond)  "<vehAssociatedSysStateSuppCond>"
  Bus Creator1/1 -> Bus Selector/1, Bus Selector1/1
  AEB_PedResp/1 -> Bus Creator1/1  "AEB_PedResp"
  blockTimeBrakeReady/1 -> Bus Creator1/6  "blockTimeBrakeReady"
  FCW_AEB_JAFctSts/1 -> Bus Creator1/2  "FCW_AEB_JAFctSts"
  "VehicleConfigAEBPed "/1 -> Bus Creator1/5  "VehicleConfigAEBPed"
  vehUnstableStateFlg/1 -> Bus Creator1/3  "vehUnstableStateFlg"
  vehAssociatedSysStateSuppCond/1 -> Bus Creator1/4  "vehAssociatedSysStateSuppCond"
  VehStateCalcJAPrefillSuppCalc/1(VSC_JACycPrefillSuppCond) -> VSC_JACycPrefillSuppCond/1
  VehStateCalcJAPrefillAborCalc/1(VSC_JACycPrefillAborCond) -> VSC_JACycPrefillAborCond/1

== system TosAeb/FcwAebJa_Step_sys/ALG_AEBSystem/ALG_AEBController/AEB_VehicleStateCalcApp/JaSuppAborCondtionCalc/JAPedAEBSuppAborConditonCalc ==
  AEB_PedResp : Inport
  FCW_AEB_JAFctSts : Inport  Port=2
  vehUnstableStateFlg : Inport  Port=3
  vehAssociatedSysStateSuppCond : Inport  Port=4
  "VehicleConfigAEBPed " : Inport  Port=5
  blockTimeBrake : Inport  Port=6
  Bus Creator : BusCreator  Inputs=6
  Bus Selector : BusSelector  OutputSignals=AEB_PedResp,FCW_AEB_JAFctSts,vehUnstableStateFlg,vehAssociatedSysStateSuppCond,VehicleConfigAEBPed,blockTimeBrake
  Bus Selector1 : BusSelector  OutputSignals=AEB_PedResp,FCW_AEB_JAFctSts,vehAssociatedSysStateSuppCond,vehUnstableStateFlg
  VehStateCalcJAAEBAborCalc : Subsystem
  VehStateCalcJAAEBSuppCalc : Subsystem
  VSC_JAPedAEBfullSuppCond : Outport
  VSC_JAPedAEBfullAborCond : Outport  Port=2
  wires:
  VehStateCalcJAAEBAborCalc/1(VSCJAPedAEBfullAborCond) -> VSC_JAPedAEBfullAborCond/1
  VehStateCalcJAAEBSuppCalc/1(VSCJAPedAEBfullSuppCond) -> VSC_JAPedAEBfullSuppCond/1
  blockTimeBrake/1 -> Bus Creator/6  "blockTimeBrake"
  "VehicleConfigAEBPed "/1 -> Bus Creator/5  "VehicleConfigAEBPed"
  vehAssociatedSysStateSuppCond/1 -> Bus Creator/4  "vehAssociatedSysStateSuppCond"
  vehUnstableStateFlg/1 -> Bus Creator/3  "vehUnstableStateFlg"
  FCW_AEB_JAFctSts/1 -> Bus Creator/2  "FCW_AEB_JAFctSts"
  AEB_PedResp/1 -> Bus Creator/1  "AEB_PedResp"
  Bus Creator/1 -> Bus Selector1/1, Bus Selector/1
  Bus Selector/4 -> VehStateCalcJAAEBSuppCalc/4(vehAssociatedSysStateSuppCond)  "<vehAssociatedSysStateSuppCond>"
  Bus Selector/3 -> VehStateCalcJAAEBSuppCalc/3(vehUnstableStateFlg)  "<vehUnstableStateFlg>"
  Bus Selector/5 -> VehStateCalcJAAEBSuppCalc/5("VehicleConfigAEBPed ")  "<VehicleConfigAEBPed>"
  Bus Selector/2 -> VehStateCalcJAAEBSuppCalc/2(FCW_AEB_JAFctSts)  "<FCW_AEB_JAFctSts>"
  Bus Selector/6 -> VehStateCalcJAAEBSuppCalc/6(blockTimeBrake)  "<blockTimeBrake>"
  Bus Selector/1 -> VehStateCalcJAAEBSuppCalc/1(AEB_PedResp)  "<AEB_PedResp>"
  Bus Selector1/1 -> VehStateCalcJAAEBAborCalc/1(AEB_PedResp)  "<AEB_PedResp>"
  Bus Selector1/2 -> VehStateCalcJAAEBAborCalc/2(FCW_AEB_JAFctSts)  "<FCW_AEB_JAFctSts>"
  Bus Selector1/3 -> VehStateCalcJAAEBAborCalc/3(vehAssociatedSysStateSuppCond)  "<vehAssociatedSysStateSuppCond>"
  Bus Selector1/4 -> VehStateCalcJAAEBAborCalc/4(vehUnstableStateFlg)  "<vehUnstableStateFlg>"

== system TosAeb/FcwAebJa_Step_sys/ALG_AEBSystem/ALG_AEBController/AEB_VehicleStateCalcApp/JaSuppAborCondtionCalc/JAPedPrefillSuppAborConditonCalc ==
  AEB_PedResp : Inport
  FCW_AEB_JAFctSts : Inport  Port=2
  vehUnstableStateFlg : Inport  Port=3
  vehAssociatedSysStateSuppCond : Inport  Port=4
  "VehicleConfigAEBPed " : Inport  Port=5
  blockTimeBrakeReady : Inport  Port=6
  Bus Creator1 : BusCreator  Inputs=6
  Bus Selector : BusSelector  OutputSignals=AEB_PedResp,FCW_AEB_JAFctSts,vehUnstableStateFlg,vehAssociatedSysStateSuppCond,VehicleConfigAEBPed,blockTimeBrakeReady
  Bus Selector1 : BusSelector  OutputSignals=AEB_PedResp,FCW_AEB_JAFctSts,vehAssociatedSysStateSuppCond,vehUnstableStateFlg
  VehStateCalcJAPrefillAborCalc : Subsystem
  VehStateCalcJAPrefillSuppCalc : Subsystem
  VSC_JAPedPrefillSuppCond : Outport
  VSC_JAPedPrefillAborCond : Outport  Port=2
  wires:
  VehStateCalcJAPrefillAborCalc/1(VSCJAPedPrefillAborCond) -> VSC_JAPedPrefillAborCond/1
  VehStateCalcJAPrefillSuppCalc/1(VSCJAPedPrefillSuppCond) -> VSC_JAPedPrefillSuppCond/1
  vehAssociatedSysStateSuppCond/1 -> Bus Creator1/4  "vehAssociatedSysStateSuppCond"
  vehUnstableStateFlg/1 -> Bus Creator1/3  "vehUnstableStateFlg"
  "VehicleConfigAEBPed "/1 -> Bus Creator1/5  "VehicleConfigAEBPed"
  FCW_AEB_JAFctSts/1 -> Bus Creator1/2  "FCW_AEB_JAFctSts"
  blockTimeBrakeReady/1 -> Bus Creator1/6  "blockTimeBrakeReady"
  AEB_PedResp/1 -> Bus Creator1/1  "AEB_PedResp"
  Bus Creator1/1 -> Bus Selector1/1, Bus Selector/1
  Bus Selector/4 -> VehStateCalcJAPrefillSuppCalc/4(vehAssociatedSysStateSuppCond)  "<vehAssociatedSysStateSuppCond>"
  Bus Selector/3 -> VehStateCalcJAPrefillSuppCalc/3(vehUnstableStateFlg)  "<vehUnstableStateFlg>"
  Bus Selector/5 -> VehStateCalcJAPrefillSuppCalc/5("VehicleConfigAEBPed ")  "<VehicleConfigAEBPed>"
  Bus Selector/2 -> VehStateCalcJAPrefillSuppCalc/2(FCW_AEB_JAFctSts)  "<FCW_AEB_JAFctSts>"
  Bus Selector/6 -> VehStateCalcJAPrefillSuppCalc/6(blockTimeBrakeReady)  "<blockTimeBrakeReady>"
  Bus Selector/1 -> VehStateCalcJAPrefillSuppCalc/1(AEB_PedResp)  "<AEB_PedResp>"
  Bus Selector1/1 -> VehStateCalcJAPrefillAborCalc/1(AEB_PedResp)  "<AEB_PedResp>"
  Bus Selector1/2 -> VehStateCalcJAPrefillAborCalc/2(FCW_AEB_JAFctSts)  "<FCW_AEB_JAFctSts>"
  Bus Selector1/3 -> VehStateCalcJAPrefillAborCalc/3(vehAssociatedSysStateSuppCond)  "<vehAssociatedSysStateSuppCond>"
  Bus Selector1/4 -> VehStateCalcJAPrefillAborCalc/4(vehUnstableStateFlg)  "<vehUnstableStateFlg>"

== system TosAeb/FcwAebJa_Step_sys/ALG_AEBSystem/ALG_AEBController/AEB_VehicleStateCalcApp/JaSuppAborCondtionCalc/JAVehAEBSuppAborConditonCalc ==
  AEB_JAResp : Inport
  FCW_AEB_JAFctSts : Inport  Port=2
  vehUnstableStateFlg : Inport  Port=3
  vehAssociatedSysStateSuppCond : Inport  Port=4
  "vehConfigAEBJA " : Inport  Port=5
  blockTimeBrake : Inport  Port=6
  Bus Creator : BusCreator  Inputs=6
  Bus Selector : BusSelector  OutputSignals=AEB_JAResp,FCW_AEB_JAFctSts,vehUnstableSts,vehAssociatedSysStsSuppFlg,VehConfigAEBJA,blockTimeBrake
  Bus Selector1 : BusSelector  OutputSignals=AEB_JAResp,FCW_AEB_JAFctSts,vehAssociatedSysStsSuppFlg,vehUnstableSts
  VehStateCalcJAAEBAborCalc : Subsystem
  VehStateCalcJAAEBSuppCalc : Subsystem
  VSC_JAVehAEBfullSuppCond : Outport
  VSC_JAVehAEBAborCond : Outport  Port=2
  wires:
  VehStateCalcJAAEBAborCalc/1(VSCJAVehAEBAborCond) -> VSC_JAVehAEBAborCond/1
  VehStateCalcJAAEBSuppCalc/1(VSCJAVehAEBfullSuppCond) -> VSC_JAVehAEBfullSuppCond/1
  Bus Selector1/4 -> VehStateCalcJAAEBAborCalc/4(vehUnstableStateFlg)  "<vehUnstableSts>"
  Bus Selector1/3 -> VehStateCalcJAAEBAborCalc/3(vehAssociatedSysStateSuppCond)  "<vehAssociatedSysStsSuppFlg>"
  Bus Selector1/2 -> VehStateCalcJAAEBAborCalc/2(FCW_AEB_JAFctSts)  "<FCW_AEB_JAFctSts>"
  Bus Selector1/1 -> VehStateCalcJAAEBAborCalc/1(VSCAEB_JAResp)  "<AEB_JAResp>"
  Bus Selector/1 -> VehStateCalcJAAEBSuppCalc/1(VSCAEB_JAResp)  "<AEB_JAResp>"
  Bus Selector/6 -> VehStateCalcJAAEBSuppCalc/6(blockTimeBrake)  "<blockTimeBrake>"
  Bus Selector/2 -> VehStateCalcJAAEBSuppCalc/2(FCW_AEB_JAFctSts)  "<FCW_AEB_JAFctSts>"
  Bus Selector/5 -> VehStateCalcJAAEBSuppCalc/5("vehConfigAEBJA ")  "<VehConfigAEBJA>"
  Bus Selector/3 -> VehStateCalcJAAEBSuppCalc/3(vehUnstableStateFlg)  "<vehUnstableSts>"
  Bus Selector/4 -> VehStateCalcJAAEBSuppCalc/4(vehAssociatedSysStateSuppCond)  "<vehAssociatedSysStsSuppFlg>"
  Bus Creator/1 -> Bus Selector/1, Bus Selector1/1
  AEB_JAResp/1 -> Bus Creator/1
  FCW_AEB_JAFctSts/1 -> Bus Creator/2
  vehUnstableStateFlg/1 -> Bus Creator/3
  vehAssociatedSysStateSuppCond/1 -> Bus Creator/4
  "vehConfigAEBJA "/1 -> Bus Creator/5
  blockTimeBrake/1 -> Bus Creator/6

== system TosAeb/FcwAebJa_Step_sys/ALG_AEBSystem/ALG_AEBController/AEB_VehicleStateCalcApp/JaSuppAborCondtionCalc/JAVehPrefillSuppAborConditonCalc ==
  AEB_JAResp : Inport
  FCW_AEB_JAFctSts : Inport  Port=2
  vehUnstableStateFlg : Inport  Port=3
  vehAssociatedSysStateSuppCond : Inport  Port=4
  "VehConfigAEBJA " : Inport  Port=5
  blockTimeBrakeReady : Inport  Port=6
  Bus Creator1 : BusCreator  Inputs=6
  Bus Selector : BusSelector  OutputSignals=AEB_JAResp,FCW_AEB_JAFctSts,vehUnstableSts,vehAssociatedSysStsSuppFlg,VehConfigAEBJA,blockTimeBrakeReady
  Bus Selector1 : BusSelector  OutputSignals=AEB_JAResp,FCW_AEB_JAFctSts,vehAssociatedSysStsSuppFlg,vehUnstableSts
  VehStateCalcJAPrefillAborCalc : Subsystem
  VehStateCalcJAPrefillSuppCalc : Subsystem
  VSC_JAVehPrefillSuppCond : Outport
  VSC_JAVehPrefillAborCond : Outport  Port=2
  wires:
  VehStateCalcJAPrefillAborCalc/1(VSCJAVehPrefillAborCond) -> VSC_JAVehPrefillAborCond/1
  VehStateCalcJAPrefillSuppCalc/1(VSCJAVehPrefillSuppCond) -> VSC_JAVehPrefillSuppCond/1
  Bus Selector1/4 -> VehStateCalcJAPrefillAborCalc/4(vehUnstableStateFlg)  "<vehUnstableSts>"
  Bus Selector1/3 -> VehStateCalcJAPrefillAborCalc/3(vehAssociatedSysStateSuppCond)  "<vehAssociatedSysStsSuppFlg>"
  Bus Selector1/2 -> VehStateCalcJAPrefillAborCalc/2(FCW_AEB_JAFctSts)  "<FCW_AEB_JAFctSts>"
  Bus Selector1/1 -> VehStateCalcJAPrefillAborCalc/1(AEB_JAResp)  "<AEB_JAResp>"
  Bus Selector/1 -> VehStateCalcJAPrefillSuppCalc/1(AEB_JAResp)  "<AEB_JAResp>"
  Bus Selector/6 -> VehStateCalcJAPrefillSuppCalc/6(blockTimeBrakeReady)  "<blockTimeBrakeReady>"
  Bus Selector/2 -> VehStateCalcJAPrefillSuppCalc/2(FCW_AEB_JAFctSts)  "<FCW_AEB_JAFctSts>"
  Bus Selector/5 -> VehStateCalcJAPrefillSuppCalc/5("VehConfigAEBJA ")  "<VehConfigAEBJA>"
  Bus Selector/3 -> VehStateCalcJAPrefillSuppCalc/3(vehUnstableStateFlg)  "<vehUnstableSts>"
  Bus Selector/4 -> VehStateCalcJAPrefillSuppCalc/4(vehAssociatedSysStateSuppCond)  "<vehAssociatedSysStsSuppFlg>"
  Bus Creator1/1 -> Bus Selector/1, Bus Selector1/1
  AEB_JAResp/1 -> Bus Creator1/1
  blockTimeBrakeReady/1 -> Bus Creator1/6
  FCW_AEB_JAFctSts/1 -> Bus Creator1/2
  "VehConfigAEBJA "/1 -> Bus Creator1/5
  vehUnstableStateFlg/1 -> Bus Creator1/3
  vehAssociatedSysStateSuppCond/1 -> Bus Creator1/4

== system TosAeb/FcwAebJa_Step_sys/ALG_AEBSystem/ALG_AEBController/AEB_VehicleStateCalcApp/PedSuppAborCondtionCalc/PedABASuppAborConditonCalc ==
  AEB_PedResp : Inport
  FCW_AEB_PedFctSts : Inport  Port=2
  vehUnstableStateFlg : Inport  Port=3
  vehAssociatedSysStateSuppCond : Inport  Port=4
  "vehConfigAEBPed " : Inport  Port=5
  blockTimeBrakeReady : Inport  Port=6
  Bus Creator : BusCreator  Inputs=6
  Bus Selector : BusSelector  OutputSignals=AEB_PedResp,FCW_AEB_PedFctSts,vehUnstableSts,vehAssociatedSysStsSuppFlg,VehicleConfigAEBPed,blockTimeBrakeReady
  Bus Selector1 : BusSelector  OutputSignals=AEB_PedResp,FCW_AEB_PedFctSts
  VehStateCalcPedABAAborCalc : Subsystem
  VehStateCalcPedABASuppCalc : Subsystem
  VSCPedABASuppCond : Outport
  VSCPedABAAborCond : Outport  Port=2
  wires:
  VehStateCalcPedABAAborCalc/1(VSCPedABAAborCond) -> VSCPedABAAborCond/1  "pedABAAborFlg"
  VehStateCalcPedABASuppCalc/1(VSCPedABASuppCond) -> VSCPedABASuppCond/1  "pedABASuppFlg"
  Bus Selector1/1 -> VehStateCalcPedABAAborCalc/1(AEB_PedResp)  "<AEB_PedResp>"
  Bus Selector1/2 -> VehStateCalcPedABAAborCalc/2(FCW_AEB_PedFctSts)  "<FCW_AEB_PedFctSts>"
  Bus Selector/1 -> VehStateCalcPedABASuppCalc/1(VSCAEB_PedResp)  "<AEB_PedResp>"
  Bus Selector/6 -> VehStateCalcPedABASuppCalc/6(blockTimeBrakeReady)  "<blockTimeBrakeReady>"
  Bus Selector/2 -> VehStateCalcPedABASuppCalc/2(FCW_AEB_PedFctSts)  "<FCW_AEB_PedFctSts>"
  Bus Selector/5 -> VehStateCalcPedABASuppCalc/5("vehConfigAEBPed ")  "<VehicleConfigAEBPed>"
  Bus Selector/3 -> VehStateCalcPedABASuppCalc/3(vehUnstableStateFlg)  "<vehUnstableSts>"
  Bus Selector/4 -> VehStateCalcPedABASuppCalc/4(vehAssociatedSysStateSuppCond)  "<vehAssociatedSysStsSuppFlg>"
  Bus Creator/1 -> Bus Selector1/1, Bus Selector/1
  blockTimeBrakeReady/1 -> Bus Creator/6
  "vehConfigAEBPed "/1 -> Bus Creator/5
  AEB_PedResp/1 -> Bus Creator/1
  vehAssociatedSysStateSuppCond/1 -> Bus Creator/4
  FCW_AEB_PedFctSts/1 -> Bus Creator/2
  vehUnstableStateFlg/1 -> Bus Creator/3

== system TosAeb/FcwAebJa_Step_sys/ALG_AEBSystem/ALG_AEBController/AEB_VehicleStateCalcApp/PedSuppAborCondtionCalc/PedAEBfullSuppAborConditonCalc ==
  AEB_PedResp : Inport
  FCW_AEB_PedFctSts : Inport  Port=2
  vehUnstableStateFlg : Inport  Port=3
  vehAssociatedSysStateSuppCond : Inport  Port=4
  "vehConfigAEBPed " : Inport  Port=5
  blockTimeBrake : Inport  Port=6
  Bus Creator : BusCreator  Inputs=6
  Bus Selector : BusSelector  OutputSignals=AEB_PedResp,FCW_AEB_PedFctSts,vehUnstableSts,vehAssociatedSysStsSuppFlg,VehicleConfigAEBPed,blockTimeBrake
  Bus Selector1 : BusSelector  OutputSignals=AEB_PedResp,FCW_AEB_PedFctSts,vehUnstableSts,vehAssociatedSysStsSuppFlg
  VehStateCalcPedAEBfullAborCalc : Subsystem
  VehStateCalcPedAEBfullSuppCalc : Subsystem
  VSCPedAEBfullSuppCond : Outport
  VSCPedAEBfullAborCond : Outport  Port=2
  wires:
  VehStateCalcPedAEBfullAborCalc/1(VSCPedAEBfullAborCond) -> VSCPedAEBfullAborCond/1
  VehStateCalcPedAEBfullSuppCalc/1(VSCPedAEBfullSuppCond) -> VSCPedAEBfullSuppCond/1
  Bus Selector1/4 -> VehStateCalcPedAEBfullAborCalc/4(vehUnstableStateFlg)  "<vehAssociatedSysStsSuppFlg>"
  Bus Selector1/3 -> VehStateCalcPedAEBfullAborCalc/3(vehAssociatedSysStateSuppCond)  "<vehUnstableSts>"
  Bus Selector1/2 -> VehStateCalcPedAEBfullAborCalc/2(FCW_AEB_PedFctSts)  "<FCW_AEB_PedFctSts>"
  Bus Selector1/1 -> VehStateCalcPedAEBfullAborCalc/1(AEB_PedResp)  "<AEB_PedResp>"
  Bus Selector/1 -> VehStateCalcPedAEBfullSuppCalc/1(AEB_PedResp)  "<AEB_PedResp>"
  Bus Selector/6 -> VehStateCalcPedAEBfullSuppCalc/6(blockTimeBrake)  "<blockTimeBrake>"
  Bus Selector/2 -> VehStateCalcPedAEBfullSuppCalc/2(FCW_AEB_PedFctSts)  "<FCW_AEB_PedFctSts>"
  Bus Selector/5 -> VehStateCalcPedAEBfullSuppCalc/5("vehConfigAEBPed ")  "<VehicleConfigAEBPed>"
  Bus Selector/3 -> VehStateCalcPedAEBfullSuppCalc/3(vehUnstableStateFlg)  "<vehUnstableSts>"
  Bus Selector/4 -> VehStateCalcPedAEBfullSuppCalc/4(vehAssociatedSysStateSuppCond)  "<vehAssociatedSysStsSuppFlg>"
  Bus Creator/1 -> Bus Selector1/1, Bus Selector/1
  AEB_PedResp/1 -> Bus Creator/1
  blockTimeBrake/1 -> Bus Creator/6
  FCW_AEB_PedFctSts/1 -> Bus Creator/2
  "vehConfigAEBPed "/1 -> Bus Creator/5
  vehUnstableStateFlg/1 -> Bus Creator/3
  vehAssociatedSysStateSuppCond/1 -> Bus Creator/4

== system TosAeb/FcwAebJa_Step_sys/ALG_AEBSystem/ALG_AEBController/AEB_VehicleStateCalcApp/PedSuppAborCondtionCalc/PedPreWaringSuppAborConditonCalc ==
  AEB_PedResp : Inport
  FCW_AEB_PedFctSts : Inport  Port=2
  vehUnstableStateFlg : Inport  Port=3
  vehAssociatedSysStateSuppCond : Inport  Port=4
  "vehConfigAEBPed " : Inport  Port=5
  blockTimeWarning : Inport  Port=6
  Bus Creator : BusCreator  Inputs=6
  Bus Selector : BusSelector  OutputSignals=AEB_PedResp,FCW_AEB_PedFctSts,vehUnstableSts,vehAssociatedSysStsSuppFlg,VehicleConfigAEBPed,blockTimeWarning
  Bus Selector1 : BusSelector  OutputSignals=AEB_PedResp,FCW_AEB_PedFctSts,vehAssociatedSysStsSuppFlg
  VehStateCalcPedPreWaringAborCalc : Subsystem
  VehStateCalcPedPreWaringSuppCalc : Subsystem
  VSCPedPreWaringSuppCond : Outport
  VSCPedPreWaringAborCond : Outport  Port=2
  wires:
  VehStateCalcPedPreWaringAborCalc/1(VSCPedPreWaringAborCond) -> VSCPedPreWaringAborCond/1
  VehStateCalcPedPreWaringSuppCalc/1(VSCPedPreWaringSuppCond) -> VSCPedPreWaringSuppCond/1
  Bus Selector1/3 -> VehStateCalcPedPreWaringAborCalc/3(vehAssociatedSysStateSuppCond)  "<vehAssociatedSysStsSuppFlg>"
  Bus Selector1/2 -> VehStateCalcPedPreWaringAborCalc/2(FCW_AEB_PedFctSts)  "<FCW_AEB_PedFctSts>"
  Bus Selector1/1 -> VehStateCalcPedPreWaringAborCalc/1(VSCAEB_PedResp)  "<AEB_PedResp>"
  Bus Selector/6 -> VehStateCalcPedPreWaringSuppCalc/6(blockTimeWarning)  "<blockTimeWarning>"
  Bus Selector/5 -> VehStateCalcPedPreWaringSuppCalc/5("vehConfigAEBPed ")  "<VehicleConfigAEBPed>"
  Bus Selector/4 -> VehStateCalcPedPreWaringSuppCalc/4(vehAssociatedSysStateSuppCond)  "<vehAssociatedSysStsSuppFlg>"
  Bus Selector/3 -> VehStateCalcPedPreWaringSuppCalc/3(vehUnstableStateFlg)  "<vehUnstableSts>"
  Bus Selector/2 -> VehStateCalcPedPreWaringSuppCalc/2(FCW_AEB_PedFctSts)  "<FCW_AEB_PedFctSts>"
  Bus Selector/1 -> VehStateCalcPedPreWaringSuppCalc/1(AEB_PedResp)  "<AEB_PedResp>"
  Bus Creator/1 -> Bus Selector/1, Bus Selector1/1
  blockTimeWarning/1 -> Bus Creator/6
  AEB_PedResp/1 -> Bus Creator/1
  "vehConfigAEBPed "/1 -> Bus Creator/5
  FCW_AEB_PedFctSts/1 -> Bus Creator/2
  vehAssociatedSysStateSuppCond/1 -> Bus Creator/4
  vehUnstableStateFlg/1 -> Bus Creator/3

== system TosAeb/FcwAebJa_Step_sys/ALG_AEBSystem/ALG_AEBController/AEB_VehicleStateCalcApp/PedSuppAborCondtionCalc/PedPrefillSuppAborConditonCalc ==
  AEB_PedResp : Inport
  FCW_AEB_PedFctSts : Inport  Port=2
  vehUnstableStateFlg : Inport  Port=3
  vehAssociatedSysStateSuppCond : Inport  Port=4
  "vehConfigAEBPed " : Inport  Port=5
  blockTimeBrakeReady : Inport  Port=6
  Bus Creator : BusCreator  Inputs=6
  Bus Selector : BusSelector  OutputSignals=AEB_PedResp,FCW_AEB_PedFctSts,vehUnstableSts,vehAssociatedSysStsSuppFlg,VehicleConfigAEBPed,blockTimeBrakeReady
  Bus Selector1 : BusSelector  OutputSignals=AEB_PedResp,FCW_AEB_PedFctSts,vehUnstableSts,vehAssociatedSysStsSuppFlg
  VehStateCalcPrefillAborCalc : Subsystem
  VehStateCalcPrefillSuppCalc : Subsystem
  VSCPedPrefillSuppCond : Outport
  VSCPedPrefillAborCond : Outport  Port=2
  wires:
  VehStateCalcPrefillAborCalc/1(VSCPedPrefillAborCond) -> VSCPedPrefillAborCond/1
  VehStateCalcPrefillSuppCalc/1(VSCPedPrefillSuppCond) -> VSCPedPrefillSuppCond/1
  Bus Selector1/4 -> VehStateCalcPrefillAborCalc/4(vehAssociatedSysStateSuppCond)  "<vehAssociatedSysStsSuppFlg>"
  Bus Selector1/3 -> VehStateCalcPrefillAborCalc/3(vehUnstableStateFlg)  "<vehUnstableSts>"
  Bus Selector1/2 -> VehStateCalcPrefillAborCalc/2(FCW_AEB_PedFctSts)  "<FCW_AEB_PedFctSts>"
  Bus Selector1/1 -> VehStateCalcPrefillAborCalc/1(AEB_PedResp)  "<AEB_PedResp>"
  Bus Selector/6 -> VehStateCalcPrefillSuppCalc/6(blockTimeBrakeReady)  "<blockTimeBrakeReady>"
  Bus Selector/5 -> VehStateCalcPrefillSuppCalc/5("vehConfigAEBPed ")  "<VehicleConfigAEBPed>"
  Bus Selector/4 -> VehStateCalcPrefillSuppCalc/4(vehAssociatedSysStateSuppCond)  "<vehAssociatedSysStsSuppFlg>"
  Bus Selector/3 -> VehStateCalcPrefillSuppCalc/3(vehUnstableStateFlg)  "<vehUnstableSts>"
  Bus Selector/2 -> VehStateCalcPrefillSuppCalc/2(FCW_AEB_PedFctSts)  "<FCW_AEB_PedFctSts>"
  Bus Selector/1 -> VehStateCalcPrefillSuppCalc/1(AEB_PedResp)  "<AEB_PedResp>"
  Bus Creator/1 -> Bus Selector1/1, Bus Selector/1
  AEB_PedResp/1 -> Bus Creator/1
  blockTimeBrakeReady/1 -> Bus Creator/6
  FCW_AEB_PedFctSts/1 -> Bus Creator/2
  "vehConfigAEBPed "/1 -> Bus Creator/5
  vehUnstableStateFlg/1 -> Bus Creator/3
  vehAssociatedSysStateSuppCond/1 -> Bus Creator/4
